simulink model "l8_ver121"  (Simulink 7.9) (continued)
== system l8_ver121 == (continued)
      mask init: same as l8_ver121/adcsnap0
      mask StyleString: popup(bram|dram),popup(1|2|3|4),popup(200|250|266|300|333),edit,popup(8|16|32|64|128),checkbox,checkbox,checkbox,checkbox
      mask CallbackString: snapshot_callback();||||||||
  s1p2re_snap : Subsystem  AncestorBlock=casper_library_scopes/snapshot  mask(snapshot){storage=bram, dram_dimm=1, dram_clock=200, nsamples=12, data_width=8, offset=off, circap=off, value=off, use_dsp48=on}
      mask prompts: storage "Storage medium"; dram_dimm "DRAM dimm"; dram_clock "DRAM clock rate"; nsamples "Number of Samples (2^?)"; data_width "Data width"; offset "Start delay support"; circap "Circular capture support"; value "Extra value capture support"; use_dsp48 "Use DSP48s to implement counters"
      mask help:
      | Standard snap block, but with the following optional extras;
      |  1) Optionally delay the fabric trigger by a user-specified number of valids.
      |  2) Capture continuously after a trigger until a stop pulse is received. Data is captured into BRAM continuously, with old data being overwritten until a stop command is received. Then stop capturing when memory is filled. This enables you to get some data before and some data after the stop pulse. Use the 'tr_en_cnt' register to determine where you are in your vector. 'Addr' register's MSb now indicates 'busy capturing'.
      |  3) Optionally capture a value on an input port to a register at the same time as the first data sample.
      |  4) Implement counters using DSP48Es to save resources.
      mask init: same as l8_ver121/adcsnap0
      mask StyleString: popup(bram|dram),popup(1|2|3|4),popup(200|250|266|300|333),edit,popup(8|16|32|64|128),checkbox,checkbox,checkbox,checkbox
      mask CallbackString: snapshot_callback();||||||||
  s2 : Subsystem  mask{mult_latency=5, add_latency=2, bram_length=10}
      mask prompts: mult_latency "Multiplier Latency"; add_latency "Adder Latency"; bram_length "BRAM Length(2^n)"
  s3 : Subsystem  mask{mult_latency=5, add_latency=2, bram_length=10}
      mask prompts: mult_latency "Multiplier Latency"; add_latency "Adder Latency"; bram_length "BRAM Length(2^n)"
  s4 : Subsystem  mask{mult_latency=5, add_latency=2, bram_length=10}
      mask prompts: mult_latency "Multiplier Latency"; add_latency "Adder Latency"; bram_length "BRAM Length(2^n)"
  s5 : Subsystem  mask{mult_latency=5, add_latency=2, bram_length=10}
      mask prompts: mult_latency "Multiplier Latency"; add_latency "Adder Latency"; bram_length "BRAM Length(2^n)"
  s6 : Subsystem  mask{mult_latency=5, add_latency=2, bram_length=10}
      mask prompts: mult_latency "Multiplier Latency"; add_latency "Adder Latency"; bram_length "BRAM Length(2^n)"
  s7 : Subsystem  mask{mult_latency=5, add_latency=2, bram_length=10}
      mask prompts: mult_latency "Multiplier Latency"; add_latency "Adder Latency"; bram_length "BRAM Length(2^n)"
  sg : Subsystem
  ssg : Subsystem
  status : xps_library/software register  Tag=xps:sw_reg  io_dir=From Processor  arith_type=Unsigned  bitwidth=32  bin_pt=0  sample_period=1  gw_name=l8_ver121_status_user_data_out
      link override newmode13_status_user_data_out: arith_type=Unsigned  n_bits=32  bin_pt=0  period=1
      link override packetizer_status_user_data_out: arith_type=Unsigned  n_bits=32  bin_pt=0  period=1
  subband_sampler : Subsystem  mask{subband=1}
      mask prompts: subband "Sub-band number (1 - 8)"
      mask init:
      | if ((subband < 1) || (subband > 8))
      |     err_msg = sprintf('Sub-band number should be in [1, 8]!');
      |     errordlg(err_msg);
      | end
      | 
      | if (mod(subband, 2) == 1)   % odd
      |     offset = 32 * subband;
      | else                        % even
      |     offset = 32 * (subband - 2);
      | end
  trig : xps_library/software register  Tag=xps:sw_reg  io_dir=From Processor  arith_type=Unsigned  bitwidth=32  bin_pt=0  sample_period=1  gw_name=l8_ver121_trig_user_data_out
      link override cic_filter_testing_to_compile_trig_user_data_out: arith_type=Unsigned  n_bits=32  bin_pt=0  period=1
      link override newmode13_trig_user_data_out: arith_type=Unsigned  n_bits=32  bin_pt=0  period=1
  val_en : Subsystem  AncestorBlock=casper_library_scopes/snapshot  mask(snapshot){storage=bram, dram_dimm=1, dram_clock=200, nsamples=12, data_width=8, offset=off, circap=off, value=off, use_dsp48=on}
      mask prompts: storage "Storage medium"; dram_dimm "DRAM dimm"; dram_clock "DRAM clock rate"; nsamples "Number of Samples (2^?)"; data_width "Data width"; offset "Start delay support"; circap "Circular capture support"; value "Extra value capture support"; use_dsp48 "Use DSP48s to implement counters"
      mask help:
      | Standard snap block, but with the following optional extras;
      |  1) Optionally delay the fabric trigger by a user-specified number of valids.
      |  2) Capture continuously after a trigger until a stop pulse is received. Data is captured into BRAM continuously, with old data being overwritten until a stop command is received. Then stop capturing when memory is filled. This enables you to get some data before and some data after the stop pulse. Use the 'tr_en_cnt' register to determine where you are in your vector. 'Addr' register's MSb now indicates 'busy capturing'.
      |  3) Optionally capture a value on an input port to a register at the same time as the first data sample.
      |  4) Implement counters using DSP48Es to save resources.
      mask init: same as l8_ver121/adcsnap0
      mask StyleString: popup(bram|dram),popup(1|2|3|4),popup(200|250|266|300|333),edit,popup(8|16|32|64|128),checkbox,checkbox,checkbox,checkbox
      mask CallbackString: snapshot_callback();||||||||
  valid_gen : Subsystem
  wires:
  Delay54/1 -> [pol1_a0]
  Delay/1 -> converter/1(u8_0)
  Delay2/1 -> converter1/1(u8_0)
  Delay3/1 -> converter2/1(u8_0)
  Delay4/1 -> converter3/1(u8_0)
  Delay5/1 -> converter4/1(u8_0)
  Delay6/1 -> converter5/1(u8_0)
  Delay7/1 -> converter6/1(u8_0)
  Delay8/1 -> converter7/1(u8_0)
  converter/1(s8_7) -> Delay54/1
  converter1/1(s8_7) -> Delay67/1
  converter2/1(s8_7) -> Delay68/1
  converter3/1(s8_7) -> Delay69/1
  converter4/1(s8_7) -> Delay70/1
  converter5/1(s8_7) -> Delay74/1
  converter6/1(s8_7) -> Delay75/1
  converter7/1(s8_7) -> Delay76/1
  Counter/1 -> Slice2/1
  Slice2/1 -> posedge/1
  posedge/1 -> [cic_sync]
  gbe0_overflow/1 -> Terminator5/1
  Constant1/1 -> dest_ip/1
  Constant7/1 -> dest_port/1
  dest_ip/1 -> [dest_ip]
  dest_port/1 -> [dest_port]
  [dest_ip] -> gbe0/4
  [dest_port] -> Slice4/1
  Constant2/1 -> status/1
  status/1 -> [status]
  packetizer/3(pkt_eof) -> gbe0/6, [pkt_eof]
  [overflow] -> gbe0_overflow/1
  [spead_rst] -> packetizer/1(reset)
  [status_bits] -> packetizer/2(status)
  Reinterpret8/1 -> [cic_pol1_re], Slice3/1
  Slice3/1 -> [s1_pol1_re]
  Slice5/1 -> [s1_pol1_im]
  Slice6/1 -> [s1_pol2_re]
  Slice7/1 -> [s1_pol2_im]
  reset/1 -> [reset]  "reset"
  Slice4/1 -> gbe0/5
  gbe0/6 -> Terminator4/1
  gbe0/7 -> Terminator6/1
  gbe0/8 -> Terminator7/1
  gbe0/9 -> Terminator8/1
  gbe0/10 -> Terminator11/1
  gbe0/11 -> Terminator3/1
  Constant11/1 -> gbe0/7
  gbe0/1 -> Terminator14/1
  gbe0/2 -> Terminator15/1
  gbe0/3 -> Terminator16/1
  gbe0/4 -> Terminator17/1
  gbe0/5 -> negedge_delay/1
  Constant12/1 -> gbe0/8
  gbe0/12 -> Terminator12/1
  [gbe_rst] -> gbe0/1
  negedge_delay/1 -> [overflow]
  valid_gen/1(valid) -> [valid]
  Constant10/1 -> reset/1
  Constant31/1 -> Register18/1
  sg/2(pps_out) -> Register18/3, [master_rst1]
  Register18/1 -> Inverter2/1, Inverter1/1, [data_enable]  "data_enable"
  arm/1 -> Slice8/1
  Pulse Generator/1 -> arm/1
  Inverter1/1 -> sg/2(pps_en)
  posedge1/1 -> Logical3/2
  Slice11/1 -> Logical3/1
  Slice9/1 -> Logical/2
  Logical3/1 -> Logical/3
  [arm] -> Register18/2
  [adc_sync] -> sg/1(pps)
  sg/1(sync_out) -> [sync_out]
  Inverter2/1 -> [spead_rst]  "spead_rst"
  Logical/1 -> [gbe_rst]  "gbe_rst"
  [overflow] -> posedge1/1
  [reset] -> Slice9/1, Slice11/1
  Delay21/1 -> Slice10/1
  Slice10/1 -> posedge4/1
  posedge4/1 -> [adc_sync]  "adc_sync"
  [spead_rst] -> valid_gen/2(reset)
  Slice8/1 -> posedge3/1
  posedge3/1 -> [arm]  "arm"
  [arm] -> Logical/1
  [valid] -> reshaper/1(valid)
  [data_enable] -> reshaper/2(data_enable)
  reshaper/1(data_out) -> packetizer/3(data), [reshaper_dout]
  reshaper/2(data_valid) -> packetizer/4(data_valid), [reshaper_valid]
  Reinterpret9/1 -> Slice5/1, [cic_pol1_im]
  Reinterpret10/1 -> [cic_pol2_re], Slice6/1
  Reinterpret11/1 -> Slice7/1, [cic_pol2_im]
  Constant4/1 -> trig/1
  trig/1 -> Slice19/1
  packetizer/1(pkt_data) -> gbe0/2, [pktizer_dout]
  packetizer/2(pkt_valid) -> gbe0/3, [pkt_valid]
  [s1_pol1_re] -> Concat1/1
  [s1_pol1_im] -> Concat1/2
  [s1_pol2_re] -> Concat1/3
  [s1_pol2_im] -> Concat1/4
  Concat6/1 -> Concat/1
  Concat5/1 -> Concat/2
  Concat2/1 -> Concat/3
  Concat1/1 -> Concat/4
  Delay38/1 -> [pol2_a0]
  Delay40/1 -> [pol2_a2]
  Delay41/1 -> [pol2_a4]
  Delay42/1 -> [pol2_a6]
  Delay44/1 -> [pol2_a8]
  Delay45/1 -> [pol2_a10]
  Delay46/1 -> [pol2_a12]
  Delay47/1 -> [pol2_a14]
  Delay25/1 -> converter8/1(u8_0)
  Delay33/1 -> converter9/1(u8_0)
  Delay35/1 -> converter10/1(u8_0)
  Delay36/1 -> converter11/1(u8_0)
  Delay37/1 -> converter12/1(u8_0)
  Delay39/1 -> converter13/1(u8_0)
  Delay43/1 -> converter14/1(u8_0)
  Delay48/1 -> converter15/1(u8_0)
  converter8/1(s8_7) -> Delay38/1
  converter9/1(s8_7) -> Delay40/1
  converter10/1(s8_7) -> Delay41/1
  converter11/1(s8_7) -> Delay42/1
  converter12/1(s8_7) -> Delay44/1
  converter13/1(s8_7) -> Delay45/1
  converter14/1(s8_7) -> Delay46/1
  converter15/1(s8_7) -> Delay47/1
  asiaa_adc5g/2(a1) -> [a1]
  asiaa_adc5g/4(a3) -> [a3]
  asiaa_adc5g/6(a5) -> [a5]
  asiaa_adc5g/8(a7) -> [a7]
  asiaa_adc5g/10(a9) -> [a9]
  asiaa_adc5g/12(a11) -> [a11]
  asiaa_adc5g/14(a13) -> [a13]
  asiaa_adc5g/16(a15) -> [a15]
  asiaa_adc5g/1(a0) -> [a0]
  asiaa_adc5g/15(a14) -> [a14]
  asiaa_adc5g/13(a12) -> [a12]
  asiaa_adc5g/11(a10) -> [a10]
  asiaa_adc5g/7(a6) -> [a6]
  asiaa_adc5g/5(a4) -> [a4]
  asiaa_adc5g/3(a2) -> [a2]
  asiaa_adc5g/9(a8) -> [a8]
  [a0] -> Delay/1
  [a2] -> Delay2/1
  [a4] -> Delay3/1
  [a6] -> Delay4/1
  [a8] -> Delay5/1
  [a10] -> Delay6/1
  [a12] -> Delay7/1
  [a14] -> Delay8/1
  [b0] -> Delay25/1
  [b2] -> Delay33/1
  [b4] -> Delay35/1
  [b6] -> Delay36/1
  [b8] -> Delay37/1
  [b10] -> Delay39/1
  [b12] -> Delay43/1
  [b14] -> Delay48/1
  asiaa_adc5g/17(sync_out) -> Delay21/1
  Constant5/1 -> val_en/2(we), val_en/3(trig)
  [valid] -> bus_create/1
  [data_enable] -> bus_create/2
  bus_create/1 -> val_en/1(din)
  asiaa_adc5g1/1(a0) -> [b0]
  asiaa_adc5g1/2(a1) -> [b1]
  asiaa_adc5g1/3(a2) -> [b2]
  asiaa_adc5g1/4(a3) -> [b3]
  asiaa_adc5g1/5(a4) -> [b4]
  asiaa_adc5g1/6(a5) -> [b5]
  asiaa_adc5g1/7(a6) -> [b6]
  asiaa_adc5g1/8(a7) -> [b7]
  asiaa_adc5g1/9(a8) -> [b8]
  asiaa_adc5g1/10(a9) -> [b9]
  asiaa_adc5g1/11(a10) -> [b10]
  asiaa_adc5g1/12(a11) -> [b11]
  asiaa_adc5g1/13(a12) -> [b12]
  asiaa_adc5g1/14(a13) -> [b13]
  asiaa_adc5g1/15(a14) -> [b14]
  asiaa_adc5g1/16(a15) -> [b15]
  Delay11/1 -> [pol1_a1]
  Delay9/1 -> converter16/1(u8_0)
  converter16/1(s8_7) -> Delay11/1
  [a1] -> Delay9/1
  Delay13/1 -> converter17/1(u8_0)
  converter17/1(s8_7) -> Delay12/1
  [a3] -> Delay13/1
  Delay15/1 -> converter18/1(u8_0)
  converter18/1(s8_7) -> Delay14/1
  [a5] -> Delay15/1
  Delay17/1 -> converter19/1(u8_0)
  converter19/1(s8_7) -> Delay16/1
  [a7] -> Delay17/1
  Delay26/1 -> converter20/1(u8_0)
  converter20/1(s8_7) -> Delay23/1
  [a9] -> Delay26/1
  Delay28/1 -> converter21/1(u8_0)
  converter21/1(s8_7) -> Delay27/1
  [a11] -> Delay28/1
  Delay29/1 -> converter22/1(u8_0)
  converter22/1(s8_7) -> Delay30/1
  [a13] -> Delay29/1
  Delay32/1 -> [pol2_a1]
  Delay31/1 -> converter23/1(u8_0)
  converter23/1(s8_7) -> Delay32/1
  [b1] -> Delay31/1
  Delay49/1 -> [pol2_a3]
  Delay34/1 -> converter24/1(u8_0)
  converter24/1(s8_7) -> Delay49/1
  [b3] -> Delay34/1
  Delay51/1 -> [pol2_a5]
  Delay50/1 -> converter25/1(u8_0)
  converter25/1(s8_7) -> Delay51/1
  [b5] -> Delay50/1
  Delay53/1 -> [pol2_a7]
  Delay52/1 -> converter26/1(u8_0)
  converter26/1(s8_7) -> Delay53/1
  [b7] -> Delay52/1
  Delay56/1 -> [pol2_a9]
  Delay55/1 -> converter27/1(u8_0)
  converter27/1(s8_7) -> Delay56/1
  [b9] -> Delay55/1
  Delay58/1 -> [pol2_a11]
  Delay57/1 -> converter28/1(u8_0)
  converter28/1(s8_7) -> Delay58/1
  [b11] -> Delay57/1
  Delay60/1 -> [pol2_a13]
  Delay59/1 -> converter29/1(u8_0)
  converter29/1(s8_7) -> Delay60/1
  [b13] -> Delay59/1
  Delay62/1 -> converter30/1(u8_0)
  converter30/1(s8_7) -> Delay61/1
  [a15] -> Delay62/1
  Delay63/1 -> [pol2_a15]
  Delay64/1 -> converter31/1(u8_0)
  converter31/1(s8_7) -> Delay63/1
  [b15] -> Delay64/1
  s1/2(pol1_im) -> Reinterpret9/1
  s1/1(pol1_re) -> Reinterpret8/1
  s1/3(pol2_re) -> Reinterpret10/1
  s1/4(pol2_im) -> Reinterpret11/1
  [cic_sync] -> Delay97/1
  [pol1_a0] -> Delay1/1
  Constant23/1 -> adcsnap0/2(we)
  bus_create1/1(bus_out) -> adcsnap0/1(din)
  Constant24/1 -> adcsnap1/2(we)
  bus_create2/1(bus_out) -> adcsnap1/1(din)
  [cic_pol1_re] -> cic1re_snap/1(din)
  [cic_pol1_im] -> cic1im_snap/1(din)
  [cic_pol2_re] -> cic2re_snap/1(din)
  [cic_pol2_im] -> cic2im_snap/1(din)
  Constant6/1 -> s1p1re_snap/2(we), s1p1re_snap/3(trig)
  [reshaper_valid] -> reshaper_snap/2(we)
  [pkt_valid] -> packetizer_snap/2(we)
  [pktizer_dout] -> packetizer_snap/1(din)
  [valid] -> cic1re_snap/2(we)
  [valid] -> cic1im_snap/2(we)
  [valid] -> cic2re_snap/2(we)
  [valid] -> cic2im_snap/2(we)
  [trig] -> cic1re_snap/3(trig)
  [trig] -> cic1im_snap/3(trig)
  [trig] -> cic2re_snap/3(trig)
  [trig] -> cic2im_snap/3(trig)
  [trig] -> reshaper_snap/3(trig)
  Slice19/1 -> posedge2/1
  posedge2/1 -> [trig]
  [trig] -> adcsnap0/3(trig)
  [trig] -> adcsnap1/3(trig)
  [trig] -> packetizer_snap/3(trig)
  [reshaper_dout] -> reshaper_snap/1(din)
  [s1_pol1_re] -> s1p1re_snap/1(din)
  [s1_pol1_re] -> Concat1/5
  [s1_pol1_im] -> Concat1/6
  [s1_pol2_re] -> Concat1/7
  [s1_pol2_im] -> Concat1/8
  [s1_pol1_re] -> Concat2/1
  [s1_pol1_im] -> Concat2/2
  [s1_pol2_re] -> Concat2/3
  [s1_pol2_im] -> Concat2/4
  [s1_pol1_re] -> Concat2/5
  [s1_pol1_im] -> Concat2/6
  [s1_pol2_re] -> Concat2/7
  [s1_pol2_im] -> Concat2/8
  [s1_pol1_re] -> Concat6/1
  [s1_pol1_im] -> Concat6/2
  [s1_pol2_re] -> Concat6/3
  [s1_pol2_im] -> Concat6/4
  [s1_pol1_re] -> Concat6/5
  [s1_pol1_im] -> Concat6/6
  [s1_pol2_re] -> Concat6/7
  [s1_pol2_im] -> Concat6/8
  [s1_pol1_re] -> Concat5/1
  [s1_pol1_im] -> Concat5/2
  [s1_pol2_re] -> Concat5/3
  [s1_pol2_im] -> Concat5/4
  [s1_pol1_re] -> Concat5/5
  [s1_pol1_im] -> Concat5/6
  [s1_pol2_re] -> Concat5/7
  [s1_pol2_im] -> Concat5/8
  Constant3/1 -> mode_sel/1
  mode_sel/1 -> Slice40/1
  Slice40/1 -> [mode_sel]
  [mode_sel] -> valid_gen/1(mode_sel)
  Mux/1 -> reshaper/3(data_in)
  [mode_sel] -> Mux/1
  Concat/1 -> Mux/2, subband_sampler/1(data_in)
  subband_sampler/1(data_out) -> Mux/3
  [mode_sel] -> packetizer/5(mode_sel)
  Delay67/1 -> [pol1_a2]
  Delay12/1 -> [pol1_a3]
  Delay68/1 -> [pol1_a4]
  Delay14/1 -> [pol1_a5]
  Delay69/1 -> [pol1_a6]
  Delay16/1 -> [pol1_a7]
  Delay70/1 -> [pol1_a8]
  Delay23/1 -> [pol1_a9]
  Delay74/1 -> [pol1_a10]
  Delay27/1 -> [pol1_a11]
  Delay75/1 -> [pol1_a12]
  Delay30/1 -> [pol1_a13]
  Delay76/1 -> [pol1_a14]
  Delay61/1 -> [pol1_a15]
  [pol1_a1] -> Delay10/1
  [pol1_a2] -> Delay18/1
  [pol1_a3] -> Delay19/1
  [pol1_a4] -> Delay20/1
  [pol1_a5] -> Delay22/1
  [pol1_a6] -> Delay24/1
  [pol1_a7] -> Delay65/1
  [pol1_a8] -> Delay66/1
  [pol1_a9] -> Delay71/1
  [pol1_a10] -> Delay72/1
  [pol1_a11] -> Delay73/1
  [pol1_a12] -> Delay77/1
  [pol1_a13] -> Delay78/1
  [pol1_a14] -> Delay79/1
  [pol1_a15] -> Delay80/1
  [pol2_a0] -> Delay81/1
  [pol2_a1] -> Delay82/1
  [pol2_a2] -> Delay83/1
  [pol2_a3] -> Delay84/1
  [pol2_a4] -> Delay85/1
  [pol2_a5] -> Delay86/1
  [pol2_a6] -> Delay87/1
  [pol2_a7] -> Delay88/1
  [pol2_a8] -> Delay89/1
  [pol2_a9] -> Delay90/1
  [pol2_a10] -> Delay91/1
  [pol2_a11] -> Delay92/1
  [pol2_a12] -> Delay93/1
  [pol2_a13] -> Delay94/1
  [pol2_a14] -> Delay95/1
  [pol2_a15] -> Delay96/1
  [pol1_a0] -> bus_create1/1(in1)
  [pol1_a1] -> bus_create1/2(in2)
  [pol1_a2] -> bus_create1/3(in3)
  [pol1_a3] -> bus_create1/4(in4)
  [pol1_a4] -> bus_create1/5(in5)
  [pol1_a5] -> bus_create1/6(in6)
  [pol1_a6] -> bus_create1/7(in7)
  [pol1_a7] -> bus_create1/8(in8)
  [pol1_a8] -> bus_create1/9(in9)
  [pol1_a9] -> bus_create1/10(in10)
  [pol1_a10] -> bus_create1/11(in11)
  [pol1_a11] -> bus_create1/12(in12)
  [pol1_a12] -> bus_create1/13(in13)
  [pol1_a13] -> bus_create1/14(in14)
  [pol1_a14] -> bus_create1/15(in15)
  [pol1_a15] -> bus_create1/16(in16)
  [pol2_a0] -> bus_create2/1(in1)
  [pol2_a1] -> bus_create2/2(in2)
  [pol2_a2] -> bus_create2/3(in3)
  [pol2_a3] -> bus_create2/4(in4)
  [pol2_a4] -> bus_create2/5(in5)
  [pol2_a5] -> bus_create2/6(in6)
  [pol2_a6] -> bus_create2/7(in7)
  [pol2_a7] -> bus_create2/8(in8)
  [pol2_a8] -> bus_create2/9(in9)
  [pol2_a9] -> bus_create2/10(in10)
  [pol2_a10] -> bus_create2/11(in11)
  [pol2_a11] -> bus_create2/12(in12)
  [pol2_a12] -> bus_create2/13(in13)
  [pol2_a13] -> bus_create2/14(in14)
  [pol2_a14] -> bus_create2/15(in15)
  [pol2_a15] -> bus_create2/16(in16)
  ssg/1(statusbits) -> [status_bits]
  [master_rst1] -> Register/1
  Register/1 -> ssg/1(reset)
  [spec_tick] -> ssg/2(spec_tick)
  [master_rst1] -> Counter1/1
  Counter1/1 -> Slice41/1
  Slice41/1 -> edge_detect5/1(in)
  edge_detect5/1(out) -> [spec_tick]
  Constant8/1 -> s1p1im_snap/3(trig), s1p1im_snap/2(we)
  [s1_pol1_im] -> s1p1im_snap/1(din)
  Constant9/1 -> s1p2re_snap/2(we), s1p2re_snap/3(trig)
  [s1_pol2_re] -> s1p2re_snap/1(din)
  Constant13/1 -> s1p2im_snap/3(trig), s1p2im_snap/2(we)
  [s1_pol2_im] -> s1p2im_snap/1(din)
  Reinterpret3/1 -> Slice1/1
  Slice1/1 -> [s0_pol1_re]
  Slice12/1 -> [s0_pol1_im]
  Slice13/1 -> [s0_pol2_re]
  Slice14/1 -> [s0_pol2_im]
  Reinterpret4/1 -> Slice12/1
  Reinterpret1/1 -> Slice13/1
  Reinterpret2/1 -> Slice14/1
  s0/2(pol1_im) -> Reinterpret4/1
  s0/1(pol1_re) -> Reinterpret3/1
  s0/3(pol2_re) -> Reinterpret1/1
  s0/4(pol2_im) -> Reinterpret2/1
  [pol1_a0] -> Delay98/1
  [pol1_a1] -> Delay99/1
  [pol1_a2] -> Delay100/1
  [pol1_a3] -> Delay101/1
  [pol1_a4] -> Delay102/1
  [pol1_a5] -> Delay103/1
  [pol1_a6] -> Delay104/1
  [pol1_a7] -> Delay105/1
  [pol1_a8] -> Delay106/1
  [pol1_a9] -> Delay107/1
  [pol1_a10] -> Delay108/1
  [pol1_a11] -> Delay109/1
  [pol1_a12] -> Delay110/1
  [pol1_a13] -> Delay111/1
  [pol1_a14] -> Delay112/1
  [pol1_a15] -> Delay113/1
  [pol2_a0] -> Delay114/1
  [pol2_a1] -> Delay115/1
  [pol2_a2] -> Delay116/1
  [pol2_a3] -> Delay117/1
  [pol2_a4] -> Delay118/1
  [pol2_a5] -> Delay119/1
  [pol2_a6] -> Delay120/1
  [pol2_a7] -> Delay121/1
  [pol2_a8] -> Delay122/1
  [pol2_a9] -> Delay123/1
  [pol2_a10] -> Delay124/1
  [pol2_a11] -> Delay125/1
  [pol2_a12] -> Delay126/1
  [pol2_a13] -> Delay127/1
  [pol2_a14] -> Delay128/1
  [pol2_a15] -> Delay129/1
  [cic_sync] -> Delay130/1
  [pol1_a0] -> Delay131/1
  [pol1_a1] -> Delay132/1
  [pol1_a2] -> Delay133/1
  [pol1_a3] -> Delay134/1
  [pol1_a4] -> Delay135/1
  [pol1_a5] -> Delay136/1
  [pol1_a6] -> Delay137/1
  [pol1_a7] -> Delay138/1
  [pol1_a8] -> Delay139/1
  [pol1_a9] -> Delay140/1
  [pol1_a10] -> Delay141/1
  [pol1_a11] -> Delay142/1
  [pol1_a12] -> Delay144/1
  [pol1_a13] -> Delay143/1
  [pol1_a14] -> Delay145/1
  [pol1_a15] -> Delay146/1
  [pol2_a0] -> Delay147/1
  [pol2_a1] -> Delay181/1
  [pol2_a2] -> Delay182/1
  [pol2_a3] -> Delay183/1
  [pol2_a4] -> Delay184/1
  [pol2_a5] -> Delay185/1
  [pol2_a6] -> Delay186/1
  [pol2_a7] -> Delay187/1
  [pol2_a8] -> Delay188/1
  [pol2_a9] -> Delay189/1
  [pol2_a10] -> Delay190/1
  [pol2_a11] -> Delay191/1
  [pol2_a12] -> Delay192/1
  [pol2_a13] -> Delay193/1
  [pol2_a14] -> Delay194/1
  [pol2_a15] -> Delay195/1
  [cic_sync] -> Delay196/1
  Reinterpret7/1 -> Slice15/1
  Slice15/1 -> [s2_pol1_re]
  Slice16/1 -> [s2_pol1_im]
  Slice17/1 -> [s2_pol2_re]
  Slice18/1 -> [s2_pol2_im]
  Reinterpret12/1 -> Slice16/1
  Reinterpret5/1 -> Slice17/1
  Reinterpret6/1 -> Slice18/1
  s2/2(pol1_im) -> Reinterpret12/1
  s2/1(pol1_re) -> Reinterpret7/1
  s2/3(pol2_re) -> Reinterpret5/1
  s2/4(pol2_im) -> Reinterpret6/1
  Reinterpret16/1 -> Slice20/1
  Slice20/1 -> [s3_pol1_re]
  Slice21/1 -> [s3_pol1_im]
  Slice22/1 -> [s3_pol2_re]
  Slice23/1 -> [s3_pol2_im]
  Reinterpret13/1 -> Slice21/1
  Reinterpret14/1 -> Slice22/1
  Reinterpret15/1 -> Slice23/1
  s3/2(pol1_im) -> Reinterpret13/1
  s3/1(pol1_re) -> Reinterpret16/1
  s3/3(pol2_re) -> Reinterpret14/1
  s3/4(pol2_im) -> Reinterpret15/1
  [pol1_a0] -> Delay197/1
  [pol1_a1] -> Delay198/1
  [pol1_a2] -> Delay199/1
  [pol1_a3] -> Delay200/1
  [pol1_a4] -> Delay201/1
  [pol1_a5] -> Delay202/1
  [pol1_a6] -> Delay203/1
  [pol1_a7] -> Delay204/1
  [pol1_a8] -> Delay205/1
  [pol1_a9] -> Delay206/1
  [pol1_a10] -> Delay207/1
  [pol1_a11] -> Delay208/1
  [pol1_a12] -> Delay209/1
  [pol1_a13] -> Delay210/1
  [pol1_a14] -> Delay211/1
  [pol1_a15] -> Delay212/1
  [pol2_a0] -> Delay213/1
  [pol2_a1] -> Delay214/1
  [pol2_a2] -> Delay215/1
  [pol2_a3] -> Delay216/1
  [pol2_a4] -> Delay217/1
  [pol2_a5] -> Delay218/1
  [pol2_a6] -> Delay219/1
  [pol2_a7] -> Delay220/1
  [pol2_a8] -> Delay221/1
  [pol2_a9] -> Delay222/1
  [pol2_a10] -> Delay223/1
  [pol2_a11] -> Delay224/1
  [pol2_a12] -> Delay225/1
  [pol2_a13] -> Delay226/1
  [pol2_a14] -> Delay227/1
  [pol2_a15] -> Delay228/1
  [cic_sync] -> Delay229/1
  Reinterpret20/1 -> Slice24/1
  Slice24/1 -> [s4_pol1_re]
  Slice25/1 -> [s4_pol1_im]
  Slice26/1 -> [s4_pol2_re]
  Slice27/1 -> [s4_pol2_im]
  Reinterpret17/1 -> Slice25/1
  Reinterpret18/1 -> Slice26/1
  Reinterpret19/1 -> Slice27/1
  s4/2(pol1_im) -> Reinterpret17/1
  s4/1(pol1_re) -> Reinterpret20/1
  s4/3(pol2_re) -> Reinterpret18/1
  s4/4(pol2_im) -> Reinterpret19/1
  [pol1_a0] -> Delay230/1
  [pol1_a1] -> Delay231/1
  [pol1_a2] -> Delay232/1
  [pol1_a3] -> Delay233/1
  [pol1_a4] -> Delay234/1
  [pol1_a5] -> Delay235/1
  [pol1_a6] -> Delay236/1
  [pol1_a7] -> Delay237/1
  [pol1_a8] -> Delay238/1
  [pol1_a9] -> Delay239/1
  [pol1_a10] -> Delay240/1
  [pol1_a11] -> Delay241/1
  [pol1_a12] -> Delay242/1
  [pol1_a13] -> Delay243/1
  [pol1_a14] -> Delay244/1
  [pol1_a15] -> Delay245/1
  [pol2_a0] -> Delay246/1
  [pol2_a1] -> Delay247/1
  [pol2_a2] -> Delay248/1
  [pol2_a3] -> Delay249/1
  [pol2_a4] -> Delay250/1
  [pol2_a5] -> Delay251/1
  [pol2_a6] -> Delay252/1
  [pol2_a7] -> Delay253/1
  [pol2_a8] -> Delay254/1
  [pol2_a9] -> Delay255/1
  [pol2_a10] -> Delay256/1
  [pol2_a11] -> Delay257/1
  [pol2_a12] -> Delay258/1
  [pol2_a13] -> Delay259/1
  [pol2_a14] -> Delay260/1
  [pol2_a15] -> Delay261/1
  [cic_sync] -> Delay262/1
  Reinterpret24/1 -> Slice28/1
  Slice28/1 -> [s5_pol1_re]
  Slice29/1 -> [s5_pol1_im]
  Slice30/1 -> [s5_pol2_re]
  Slice31/1 -> [s5_pol2_im]
  Reinterpret21/1 -> Slice29/1
  Reinterpret22/1 -> Slice30/1
  Reinterpret23/1 -> Slice31/1
  s5/2(pol1_im) -> Reinterpret21/1
  s5/1(pol1_re) -> Reinterpret24/1
  s5/3(pol2_re) -> Reinterpret22/1
  s5/4(pol2_im) -> Reinterpret23/1
  [pol1_a0] -> Delay263/1
  [pol1_a1] -> Delay264/1
  [pol1_a2] -> Delay265/1
  [pol1_a3] -> Delay266/1
  [pol1_a4] -> Delay267/1
  [pol1_a5] -> Delay268/1
  [pol1_a6] -> Delay269/1
  [pol1_a7] -> Delay270/1
  [pol1_a8] -> Delay271/1
  [pol1_a9] -> Delay272/1
  [pol1_a10] -> Delay273/1
  [pol1_a11] -> Delay274/1
  [pol1_a12] -> Delay275/1
  [pol1_a13] -> Delay276/1
  [pol1_a14] -> Delay277/1
  [pol1_a15] -> Delay278/1
  [pol2_a0] -> Delay279/1
  [pol2_a1] -> Delay280/1
  [pol2_a2] -> Delay281/1
  [pol2_a3] -> Delay282/1
  [pol2_a4] -> Delay283/1
  [pol2_a5] -> Delay284/1
  [pol2_a6] -> Delay285/1
  [pol2_a7] -> Delay286/1
  [pol2_a8] -> Delay287/1
  [pol2_a9] -> Delay288/1
  [pol2_a10] -> Delay289/1
  [pol2_a11] -> Delay290/1
  [pol2_a12] -> Delay291/1
  [pol2_a13] -> Delay292/1
  [pol2_a14] -> Delay293/1
  [pol2_a15] -> Delay294/1
  [cic_sync] -> Delay295/1
  Reinterpret28/1 -> Slice32/1
  Slice32/1 -> [s6_pol1_re]
  Slice33/1 -> [s6_pol1_im]
  Slice34/1 -> [s6_pol2_re]
  Slice35/1 -> [s6_pol2_im]
  Reinterpret25/1 -> Slice33/1
  Reinterpret26/1 -> Slice34/1
  Reinterpret27/1 -> Slice35/1
  s6/2(pol1_im) -> Reinterpret25/1
  s6/1(pol1_re) -> Reinterpret28/1
  s6/3(pol2_re) -> Reinterpret26/1
  s6/4(pol2_im) -> Reinterpret27/1
  [pol1_a0] -> Delay296/1
  [pol1_a1] -> Delay297/1
  [pol1_a2] -> Delay298/1
  [pol1_a3] -> Delay299/1
  [pol1_a4] -> Delay300/1
  [pol1_a5] -> Delay301/1
  [pol1_a6] -> Delay302/1
  [pol1_a7] -> Delay303/1
  [pol1_a8] -> Delay304/1
  [pol1_a9] -> Delay305/1
  [pol1_a10] -> Delay306/1
  [pol1_a11] -> Delay307/1
  [pol1_a12] -> Delay308/1
  [pol1_a13] -> Delay309/1
  [pol1_a14] -> Delay310/1
  [pol1_a15] -> Delay311/1
  [pol2_a0] -> Delay312/1
  [pol2_a1] -> Delay313/1
  [pol2_a2] -> Delay314/1
  [pol2_a3] -> Delay315/1
  [pol2_a4] -> Delay316/1
  [pol2_a5] -> Delay317/1
  [pol2_a6] -> Delay318/1
  [pol2_a7] -> Delay319/1
  [pol2_a8] -> Delay320/1
  [pol2_a9] -> Delay321/1
  [pol2_a10] -> Delay322/1
  [pol2_a11] -> Delay323/1
  [pol2_a12] -> Delay324/1
  [pol2_a13] -> Delay325/1
  [pol2_a14] -> Delay326/1
  [pol2_a15] -> Delay327/1
  [cic_sync] -> Delay328/1
  Reinterpret32/1 -> Slice36/1
  Slice36/1 -> [s7_pol1_re]
  Slice37/1 -> [s7_pol1_im]
  Slice38/1 -> [s7_pol2_re]
  Slice39/1 -> [s7_pol2_im]
  Reinterpret29/1 -> Slice37/1
  Reinterpret30/1 -> Slice38/1
  Reinterpret31/1 -> Slice39/1
  s7/2(pol1_im) -> Reinterpret29/1
  s7/1(pol1_re) -> Reinterpret32/1
  s7/3(pol2_re) -> Reinterpret30/1
  s7/4(pol2_im) -> Reinterpret31/1
  Delay1/1 -> s1/1(pol1_in0)
  Delay10/1 -> s1/2(pol1_in1)
  Delay18/1 -> s1/3(pol1_in2)
  Delay19/1 -> s1/4(pol1_in3)
  Delay20/1 -> s1/5(pol1_in4)
  Delay22/1 -> s1/6(pol1_in5)
  Delay24/1 -> s1/7(pol1_in6)
  Delay65/1 -> s1/8(pol1_in7)
  Delay66/1 -> s1/9(pol1_in8)
  Delay71/1 -> s1/10(pol1_in9)
  Delay72/1 -> s1/11(pol1_in10)
  Delay73/1 -> s1/12(pol1_in11)
  Delay77/1 -> s1/13(pol1_in12)
  Delay78/1 -> s1/14(pol1_in13)
  Delay79/1 -> s1/15(pol1_in14)
  Delay80/1 -> s1/16(pol1_in15)
  Delay81/1 -> s1/17(pol2_in0)
  Delay82/1 -> s1/18(pol2_in1)
  Delay83/1 -> s1/19(pol2_in2)
  Delay84/1 -> s1/20(pol2_in3)
  Delay85/1 -> s1/21(pol2_in4)
  Delay86/1 -> s1/22(pol2_in5)
  Delay87/1 -> s1/23(pol2_in6)
  Delay88/1 -> s1/24(pol2_in7)
  Delay89/1 -> s1/25(pol2_in8)
  Delay90/1 -> s1/26(pol2_in9)
  Delay91/1 -> s1/27(pol2_in10)
  Delay92/1 -> s1/28(pol2_in11)
  Delay93/1 -> s1/29(pol2_in12)
  Delay94/1 -> s1/30(pol2_in13)
  Delay95/1 -> s1/31(pol2_in14)
  Delay96/1 -> s1/32(pol2_in15)
  Delay97/1 -> s1/33(adc_CIC_sync)
  Delay98/1 -> s0/1(pol1_in0)
  Delay99/1 -> s0/2(pol1_in1)
  Delay100/1 -> s0/3(pol1_in2)
  Delay101/1 -> s0/4(pol1_in3)
  Delay102/1 -> s0/5(pol1_in4)
  Delay103/1 -> s0/6(pol1_in5)
  Delay104/1 -> s0/7(pol1_in6)
  Delay105/1 -> s0/8(pol1_in7)
  Delay106/1 -> s0/9(pol1_in8)
  Delay107/1 -> s0/10(pol1_in9)
  Delay108/1 -> s0/11(pol1_in10)
  Delay109/1 -> s0/12(pol1_in11)
  Delay110/1 -> s0/13(pol1_in12)
  Delay111/1 -> s0/14(pol1_in13)
  Delay112/1 -> s0/15(pol1_in14)
  Delay113/1 -> s0/16(pol1_in15)
  Delay114/1 -> s0/17(pol2_in0)
  Delay115/1 -> s0/18(pol2_in1)
  Delay116/1 -> s0/19(pol2_in2)
  Delay117/1 -> s0/20(pol2_in3)
  Delay118/1 -> s0/21(pol2_in4)
  Delay119/1 -> s0/22(pol2_in5)
  Delay120/1 -> s0/23(pol2_in6)
  Delay121/1 -> s0/24(pol2_in7)
  Delay122/1 -> s0/25(pol2_in8)
  Delay123/1 -> s0/26(pol2_in9)
  Delay124/1 -> s0/27(pol2_in10)
  Delay125/1 -> s0/28(pol2_in11)
  Delay126/1 -> s0/29(pol2_in12)
  Delay127/1 -> s0/30(pol2_in13)
  Delay128/1 -> s0/31(pol2_in14)
  Delay129/1 -> s0/32(pol2_in15)
  Delay130/1 -> s0/33(adc_CIC_sync)
  Delay131/1 -> s2/1(pol1_in0)
  Delay132/1 -> s2/2(pol1_in1)
  Delay133/1 -> s2/3(pol1_in2)
  Delay134/1 -> s2/4(pol1_in3)
  Delay135/1 -> s2/5(pol1_in4)
  Delay136/1 -> s2/6(pol1_in5)
  Delay137/1 -> s2/7(pol1_in6)
  Delay138/1 -> s2/8(pol1_in7)
  Delay139/1 -> s2/9(pol1_in8)
  Delay140/1 -> s2/10(pol1_in9)
  Delay141/1 -> s2/11(pol1_in10)
  Delay142/1 -> s2/12(pol1_in11)
  Delay143/1 -> s2/14(pol1_in13)
  Delay144/1 -> s2/13(pol1_in12)
  Delay145/1 -> s2/15(pol1_in14)
  Delay146/1 -> s2/16(pol1_in15)
  Delay147/1 -> s2/17(pol2_in0)
  [pol1_a0] -> Delay148/1
  [pol1_a1] -> Delay149/1
  [pol1_a2] -> Delay150/1
  [pol1_a3] -> Delay151/1
  [pol1_a4] -> Delay152/1
  [pol1_a5] -> Delay153/1
  [pol1_a6] -> Delay154/1
  [pol1_a7] -> Delay155/1
  [pol1_a8] -> Delay156/1
  [pol1_a9] -> Delay157/1
  [pol1_a10] -> Delay158/1
  [pol1_a11] -> Delay159/1
  [pol1_a12] -> Delay161/1
  [pol1_a13] -> Delay160/1
  [pol1_a14] -> Delay162/1
  [pol1_a15] -> Delay163/1
  [pol2_a0] -> Delay164/1
  [pol2_a1] -> Delay165/1
  [pol2_a2] -> Delay166/1
  [pol2_a3] -> Delay167/1
  [pol2_a4] -> Delay168/1
  [pol2_a5] -> Delay169/1
  [pol2_a6] -> Delay170/1
  [pol2_a7] -> Delay171/1
  [pol2_a8] -> Delay172/1
  [pol2_a9] -> Delay173/1
  [pol2_a10] -> Delay174/1
  [pol2_a11] -> Delay175/1
  [pol2_a12] -> Delay177/1
  [pol2_a13] -> Delay176/1
  [pol2_a14] -> Delay178/1
  [pol2_a15] -> Delay179/1
  [cic_sync] -> Delay180/1
  Delay148/1 -> s3/1(pol1_in0)
  Delay149/1 -> s3/2(pol1_in1)
  Delay150/1 -> s3/3(pol1_in2)
  Delay151/1 -> s3/4(pol1_in3)
  Delay152/1 -> s3/5(pol1_in4)
  Delay153/1 -> s3/6(pol1_in5)
  Delay154/1 -> s3/7(pol1_in6)
  Delay155/1 -> s3/8(pol1_in7)
  Delay156/1 -> s3/9(pol1_in8)
  Delay157/1 -> s3/10(pol1_in9)
  Delay158/1 -> s3/11(pol1_in10)
  Delay159/1 -> s3/12(pol1_in11)
  Delay161/1 -> s3/13(pol1_in12)
  Delay160/1 -> s3/14(pol1_in13)
  Delay162/1 -> s3/15(pol1_in14)
  Delay163/1 -> s3/16(pol1_in15)
  Delay164/1 -> s3/17(pol2_in0)
  Delay165/1 -> s3/18(pol2_in1)
  Delay166/1 -> s3/19(pol2_in2)
  Delay167/1 -> s3/20(pol2_in3)
  Delay168/1 -> s3/21(pol2_in4)
  Delay169/1 -> s3/22(pol2_in5)
  Delay170/1 -> s3/23(pol2_in6)
  Delay171/1 -> s3/24(pol2_in7)
  Delay172/1 -> s3/25(pol2_in8)
  Delay173/1 -> s3/26(pol2_in9)
  Delay174/1 -> s3/27(pol2_in10)
  Delay175/1 -> s3/28(pol2_in11)
  Delay177/1 -> s3/29(pol2_in12)
  Delay176/1 -> s3/30(pol2_in13)
  Delay178/1 -> s3/31(pol2_in14)
  Delay179/1 -> s3/32(pol2_in15)
  Delay180/1 -> s3/33(adc_CIC_sync)
  Delay181/1 -> s2/18(pol2_in1)
  Delay182/1 -> s2/19(pol2_in2)
  Delay183/1 -> s2/20(pol2_in3)
  Delay184/1 -> s2/21(pol2_in4)
  Delay185/1 -> s2/22(pol2_in5)
  Delay186/1 -> s2/23(pol2_in6)
  Delay187/1 -> s2/24(pol2_in7)
  Delay188/1 -> s2/25(pol2_in8)
  Delay189/1 -> s2/26(pol2_in9)
  Delay190/1 -> s2/27(pol2_in10)
  Delay191/1 -> s2/28(pol2_in11)
  Delay192/1 -> s2/29(pol2_in12)
  Delay193/1 -> s2/30(pol2_in13)
  Delay194/1 -> s2/31(pol2_in14)
  Delay195/1 -> s2/32(pol2_in15)
  Delay196/1 -> s2/33(adc_CIC_sync)
  Delay197/1 -> s4/1(pol1_in0)
  Delay198/1 -> s4/2(pol1_in1)
  Delay199/1 -> s4/3(pol1_in2)
  Delay200/1 -> s4/4(pol1_in3)
  Delay201/1 -> s4/5(pol1_in4)
  Delay202/1 -> s4/6(pol1_in5)
  Delay203/1 -> s4/7(pol1_in6)
  Delay204/1 -> s4/8(pol1_in7)
  Delay205/1 -> s4/9(pol1_in8)
  Delay206/1 -> s4/10(pol1_in9)
  Delay207/1 -> s4/11(pol1_in10)
  Delay208/1 -> s4/12(pol1_in11)
  Delay209/1 -> s4/13(pol1_in12)
  Delay210/1 -> s4/14(pol1_in13)
  Delay211/1 -> s4/15(pol1_in14)
  Delay212/1 -> s4/16(pol1_in15)
  Delay213/1 -> s4/17(pol2_in0)
  Delay214/1 -> s4/18(pol2_in1)
  Delay215/1 -> s4/19(pol2_in2)
  Delay216/1 -> s4/20(pol2_in3)
  Delay217/1 -> s4/21(pol2_in4)
  Delay218/1 -> s4/22(pol2_in5)
  Delay219/1 -> s4/23(pol2_in6)
  Delay220/1 -> s4/24(pol2_in7)
  Delay221/1 -> s4/25(pol2_in8)
  Delay222/1 -> s4/26(pol2_in9)
  Delay223/1 -> s4/27(pol2_in10)
  Delay224/1 -> s4/28(pol2_in11)
  Delay225/1 -> s4/29(pol2_in12)
  Delay226/1 -> s4/30(pol2_in13)
  Delay227/1 -> s4/31(pol2_in14)
  Delay228/1 -> s4/32(pol2_in15)
  Delay229/1 -> s4/33(adc_CIC_sync)
  Delay230/1 -> s5/1(pol1_in0)
  Delay231/1 -> s5/2(pol1_in1)
  Delay232/1 -> s5/3(pol1_in2)
  Delay233/1 -> s5/4(pol1_in3)
  Delay234/1 -> s5/5(pol1_in4)
  Delay235/1 -> s5/6(pol1_in5)
  Delay236/1 -> s5/7(pol1_in6)
  Delay237/1 -> s5/8(pol1_in7)
  Delay238/1 -> s5/9(pol1_in8)
  Delay239/1 -> s5/10(pol1_in9)
  Delay240/1 -> s5/11(pol1_in10)
  Delay241/1 -> s5/12(pol1_in11)
  Delay242/1 -> s5/13(pol1_in12)
  Delay243/1 -> s5/14(pol1_in13)
  Delay244/1 -> s5/15(pol1_in14)
  Delay245/1 -> s5/16(pol1_in15)
  Delay246/1 -> s5/17(pol2_in0)
  Delay247/1 -> s5/18(pol2_in1)
  Delay248/1 -> s5/19(pol2_in2)
  Delay249/1 -> s5/20(pol2_in3)
  Delay250/1 -> s5/21(pol2_in4)
  Delay251/1 -> s5/22(pol2_in5)
  Delay252/1 -> s5/23(pol2_in6)
  Delay253/1 -> s5/24(pol2_in7)
  Delay254/1 -> s5/25(pol2_in8)
  Delay255/1 -> s5/26(pol2_in9)
  Delay256/1 -> s5/27(pol2_in10)
  Delay257/1 -> s5/28(pol2_in11)
  Delay258/1 -> s5/29(pol2_in12)
  Delay259/1 -> s5/30(pol2_in13)
  Delay260/1 -> s5/31(pol2_in14)
  Delay261/1 -> s5/32(pol2_in15)
  Delay262/1 -> s5/33(adc_CIC_sync)
  Delay263/1 -> s6/1(pol1_in0)
  Delay264/1 -> s6/2(pol1_in1)
  Delay265/1 -> s6/3(pol1_in2)
  Delay266/1 -> s6/4(pol1_in3)
  Delay267/1 -> s6/5(pol1_in4)
  Delay268/1 -> s6/6(pol1_in5)
  Delay269/1 -> s6/7(pol1_in6)
  Delay270/1 -> s6/8(pol1_in7)
  Delay271/1 -> s6/9(pol1_in8)
  Delay272/1 -> s6/10(pol1_in9)
  Delay273/1 -> s6/11(pol1_in10)
  Delay274/1 -> s6/12(pol1_in11)
  Delay275/1 -> s6/13(pol1_in12)
  Delay276/1 -> s6/14(pol1_in13)
  Delay277/1 -> s6/15(pol1_in14)
  Delay278/1 -> s6/16(pol1_in15)
  Delay279/1 -> s6/17(pol2_in0)
  Delay280/1 -> s6/18(pol2_in1)
  Delay281/1 -> s6/19(pol2_in2)
  Delay282/1 -> s6/20(pol2_in3)
  Delay283/1 -> s6/21(pol2_in4)
  Delay284/1 -> s6/22(pol2_in5)
  Delay285/1 -> s6/23(pol2_in6)
  Delay286/1 -> s6/24(pol2_in7)
  Delay287/1 -> s6/25(pol2_in8)
  Delay288/1 -> s6/26(pol2_in9)
  Delay289/1 -> s6/27(pol2_in10)
  Delay290/1 -> s6/28(pol2_in11)
  Delay291/1 -> s6/29(pol2_in12)
  Delay292/1 -> s6/30(pol2_in13)
  Delay293/1 -> s6/31(pol2_in14)
  Delay294/1 -> s6/32(pol2_in15)
  Delay295/1 -> s6/33(adc_CIC_sync)
  Delay296/1 -> s7/1(pol1_in0)
  Delay297/1 -> s7/2(pol1_in1)
  Delay298/1 -> s7/3(pol1_in2)
  Delay299/1 -> s7/4(pol1_in3)
  Delay300/1 -> s7/5(pol1_in4)
  Delay301/1 -> s7/6(pol1_in5)
  Delay302/1 -> s7/7(pol1_in6)
  Delay303/1 -> s7/8(pol1_in7)
  Delay304/1 -> s7/9(pol1_in8)
  Delay305/1 -> s7/10(pol1_in9)
  Delay306/1 -> s7/11(pol1_in10)
  Delay307/1 -> s7/12(pol1_in11)
  Delay308/1 -> s7/13(pol1_in12)
  Delay309/1 -> s7/14(pol1_in13)
  Delay310/1 -> s7/15(pol1_in14)
  Delay311/1 -> s7/16(pol1_in15)
  Delay312/1 -> s7/17(pol2_in0)
  Delay313/1 -> s7/18(pol2_in1)
  Delay314/1 -> s7/19(pol2_in2)
  Delay315/1 -> s7/20(pol2_in3)
  Delay316/1 -> s7/21(pol2_in4)
  Delay318/1 -> s7/23(pol2_in6)
  Delay317/1 -> s7/22(pol2_in5)
  Delay319/1 -> s7/24(pol2_in7)
  Delay320/1 -> s7/25(pol2_in8)
  Delay321/1 -> s7/26(pol2_in9)
  Delay322/1 -> s7/27(pol2_in10)
  Delay323/1 -> s7/28(pol2_in11)
  Delay324/1 -> s7/29(pol2_in12)
  Delay325/1 -> s7/30(pol2_in13)
  Delay326/1 -> s7/31(pol2_in14)
  Delay327/1 -> s7/32(pol2_in15)
  Delay328/1 -> s7/33(adc_CIC_sync)
